simulink model "wlan_phy_rx_pmd"  (Simulink 7.8)
settings: Solver=VariableStepDiscrete  SolverMode=SingleTasking  StartTime=0.0  StopTime=rx_sim.sim_time  RelTol=1e-3
PostLoadFcn:
  | wlan_phy_rx_init
InitFcn:
  | wlan_phy_rx_init
StartFcn:
  | wlan_phy_rx_init
values from neighbouring files (symbols this model uses):
  barker_seq20 = 0.95 * circshift(barker_seq20, [0 4]) ./ max(abs(barker_seq20))    [wlan_phy_rx_init.m]
  bit_scrambler_lfsr_bytes = sum(bit_scrambler_lfsr_bytes .* repmat(2.^[0:7], 127, 1),2)    [wlan_phy_rx_init.m]
  longCorr_coef_i = [3*real(longCorr_coef)]    [wlan_phy_rx_init.m]
  longCorr_coef_q = [3*imag(longCorr_coef)]    [wlan_phy_rx_init.m]
  MAX_CP_LEN = 32    [wlan_phy_rx_init.m]
  MAX_NCBPS = NCBPS_11n_64QAM    (= 312)    [wlan_phy_rx_init.m]
  MAX_NUM_BYTES = 2^16-1    (= 65535)    [wlan_phy_rx_init.m]
  MAX_NUM_SC = 64    [wlan_phy_rx_init.m]
  MAX_NUM_SYMS = 600    [wlan_phy_rx_init.m]
  NDBPS_11a_BPSK12 = 24    [wlan_phy_rx_init.m]
  preFFT_sampBuff_numSamps = 4*MAX_NUM_SC    (= 256)    [wlan_phy_rx_init.m]
  rx_sim = struct()    [wlan_phy_rx_init.m]
  scr_ind_rev = zeros(1,128)    [wlan_phy_rx_init.m]
undefined symbols (not defined in the file or in its folder): gcbh, mcs_rom_11ag, mcs_rom_11n, wlan_rx_deinterleave_rom_gen, xlBlockLoadCallback, xlGetNormalizedPeriod, xlipgetpartsetting
libraries: xbsIndex_r4 x4943, xbsEDKLib_r4 x1
(part 3 of 28)

== system wlan_phy_rx_pmd/FCS & Pkt Buf ==
  DSSS data : Inport
  OFDM data : Inport  Port=2
  Bus Selector : BusSelector  OutputSignals=byte_valid,byte_index,byte,last_byte
  Bus Selector1 : BusSelector  OutputSignals=byte_valid,byte_index,byte,last_byte
  Bytes In : Scope  NumInputPorts=8
      settings: TimeRange=10000  YMin=0~0~0~-1~-1~-1~-1~-1  YMax=1~90~225~1~1~1~1~1  SaveName=ScopeData36
  FCS Calc : Subsystem
  Gateway Out{1..4,8..9,11..12} (8 alike) : xbsIndex_r4/Gateway Out  inherit_from_input=off  hdl_port=off  timing_constraint=None  locs_specified=off  LOCs={}  UseAsDAC=off  DACChannel='1'  block_type=gatewayout
  Pkt Buf Interface : Subsystem
  wires:
  OFDM data/1 -> Bus Selector/1, Pkt Buf Interface/2(OFDM data), FCS Calc/2(OFDM data)
  DSSS data/1 -> Bus Selector1/1, Pkt Buf Interface/1(DSSS data), FCS Calc/1(DSSS data)
  Bus Selector1/1 -> Gateway Out9/1  "<byte_valid>"
  Bus Selector1/2 -> Gateway Out8/1  "<byte_index>"
  Bus Selector1/3 -> Gateway Out11/1  "<byte>"
  Bus Selector1/4 -> Gateway Out12/1  "<last_byte>"
  Bus Selector/1 -> Gateway Out1/1  "<byte_valid>"
  Bus Selector/2 -> Gateway Out2/1  "<byte_index>"
  Bus Selector/3 -> Gateway Out3/1  "<byte>"
  Bus Selector/4 -> Gateway Out4/1  "<last_byte>"
  Gateway Out9/1 -> Bytes In/1  "DSSS Valid"
  Gateway Out8/1 -> Bytes In/2  "DSSS Byte Index"
  Gateway Out11/1 -> Bytes In/3  "DSSS Byte"
  Gateway Out12/1 -> Bytes In/4  "DSSS Last Byte"
  Gateway Out1/1 -> Bytes In/5  "OFDM Valid"
  Gateway Out2/1 -> Bytes In/6  "OFDM Byte Index"
  Gateway Out3/1 -> Bytes In/7  "OFDM Byte"
  Gateway Out4/1 -> Bytes In/8  "OFDM Last Byte"

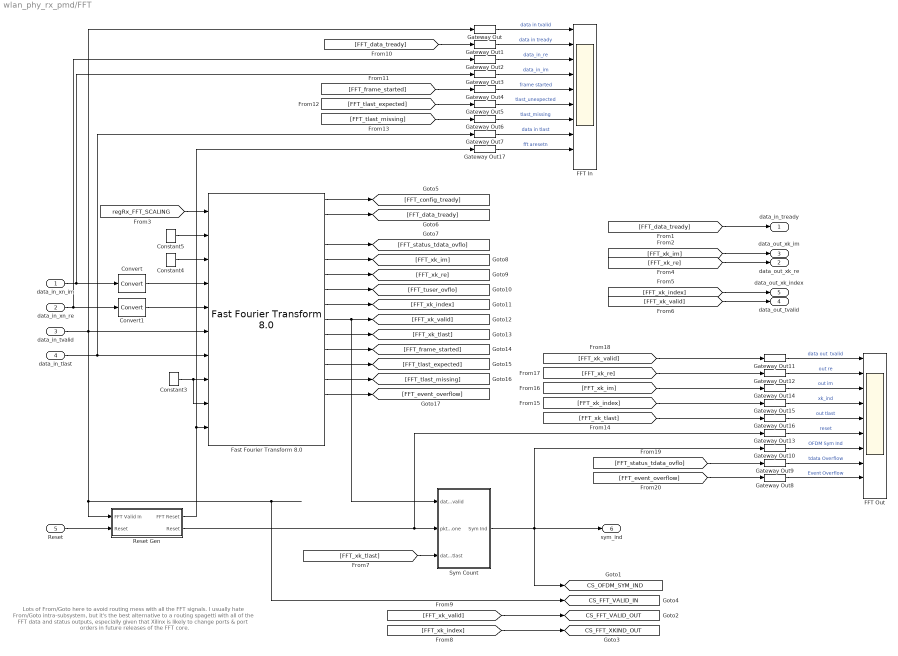
== system wlan_phy_rx_pmd/FFT ==
  note: Lots of From/Goto here to avoid routing
      | mess with all the FFT signals. I usually hate
      | From/Goto intra-subsystem, but it's the best
      | alternative to a routing spagetti with all of the
      | FFT data and status outputs, especially given
      | that Xilinx is likely to change ports & port orders
      | in future releases of the FFT core.
  data_in_xn_im : Inport
  data_in_xn_re : Inport  Port=2
  data_in_tvalid : Inport  Port=3
  data_in_tlast : Inport  Port=4
  Reset : Inport  Port=5
  Constant3 : xbsIndex_r4/Constant  const=1  gui_display_data_type=Boolean  arith_type=Boolean  n_bits=1  bin_pt=0  preci_type=Single  exp_width=8  frac_width=24  explicit_period=on  period=1  equ=P=C  opselect=C  inp2=PCIN>>17  opr=+  inp1=P  carry=CIN  dbl_ovrd=off  block_type=constant
  Constant4 : xbsIndex_r4/Constant  const=1  gui_display_data_type=Boolean  arith_type=Boolean  n_bits=1  bin_pt=0  preci_type=Single  exp_width=8  frac_width=24  explicit_period=on  period=1  equ=P=C  opselect=C  inp2=PCIN>>17  opr=+  inp1=P  carry=CIN  dbl_ovrd=off  block_type=constant
  Constant5 : xbsIndex_r4/Constant  const=1  gui_display_data_type=Fixed-point  arith_type=Unsigned  n_bits=1  bin_pt=0  preci_type=Single  exp_width=8  frac_width=24  explicit_period=on  period=1  equ=P=C  opselect=C  inp2=PCIN>>17  opr=+  inp1=P  carry=CIN  dbl_ovrd=off  block_type=constant
  Convert : xbsIndex_r4/Convert  gui_display_data_type=Fixed-point  arith_type="Signed  (2's comp)"  n_bits=24  bin_pt=23  float_type=Single  exp_bits=8  fraction_bits=24  quantization=Truncate  overflow=Wrap  en=off  latency=0  dbl_ovrd=off  pipeline=off  block_type=convert
  Convert1 : xbsIndex_r4/Convert  gui_display_data_type=Fixed-point  arith_type="Signed  (2's comp)"  n_bits=24  bin_pt=23  float_type=Single  exp_bits=8  fraction_bits=24  quantization=Truncate  overflow=Wrap  en=off  latency=0  dbl_ovrd=off  pipeline=off  block_type=convert
  FFT In : Scope  NumInputPorts=10
      settings: TimeRange=30000  YMin=-0.1~0~-0.5~-0.5~0~-1~-1~0~0~-1  YMax=1.1~1~0.5~0.5~1~1~1~1~1~1
  FFT Out : Scope  NumInputPorts=10
      settings: TimeRange=40000  YMin=0~-1~-1~0~0~0~0~0~-1~-1  YMax=1~1~1~80~1~1~40~1~1~1  SaveName=ScopeData29
  "Fast Fourier Transform 8.0 " : xbsIndex_r4/Fast Fourier Transform 8.0   transform_length=64  target_clock_frequency=160  target_data_throughput=50  implementation_options=radix_4_burst_io  run_time_configurable_transform_length=off  phase_factor_width=24  scaling_options=scaled  rounding_modes=convergent_rounding  aclken=off  aresetn=on  cyclic_prefix_insertion=off  output_ordering=natural_order  throttle_scheme=nonrealtime  xk_index=on  ovflo=on  trim_axipin_name=on  memory_options_data=block_ram  memory_options_phase_factors=block_ram  number_of_stages_using_block_ram_for_data_and_phase_factors=0  memory_options_reorder=block_ram  memory_options_hybrid=off  complex_mult_type=use_mults_performance  butterfly_type=use_luts  channels=1  input_ordering=natural_order  input_width=16  ip_name=Fast Fourier Transform  ip_version=8.0  dsptool_ready=true  ipcore_usecache=true  ipcore_useipmodelcache=true  wrapper_available=true  port_translation_map={ 'aclken' => 'en', 'aresetn' => 'rst'}  block_type=xfft_v8_0
  Gateway Out{,1..17} (18 alike) : xbsIndex_r4/Gateway Out  inherit_from_input=off  hdl_port=off  timing_constraint=None  locs_specified=off  LOCs={}  UseAsDAC=off  DACChannel='1'  block_type=gatewayout
  Reset Gen : Subsystem
  Sym Count : Subsystem
  data_in_tready : Outport
  data_out_xk_re : Outport  Port=2
  data_out_xk_im : Outport  Port=3
  data_out_tvalid : Outport  Port=4
  data_out_xk_index : Outport  Port=5
  sym_ind : Outport  Port=6
  tag scope: CS_FFT_VALID_IN=global, CS_FFT_VALID_OUT=global, CS_FFT_XKIND_OUT=global, CS_OFDM_SYM_IND=global
  wires:
  Gateway Out13/1 -> FFT Out/6  "reset"
  Gateway Out16/1 -> FFT Out/5  "out tlast"
  Gateway Out15/1 -> FFT Out/4  "xk_ind"
  Gateway Out14/1 -> FFT Out/3  "out im"
  Gateway Out12/1 -> FFT Out/2  "out re"
  Gateway Out11/1 -> FFT Out/1  "data out  tvalid"
  [FFT_xk_index] -> data_out_xk_index/1
  [FFT_xk_re] -> data_out_xk_re/1
  [FFT_xk_tlast] -> Sym Count/3(data_out_tlast)
  [FFT_xk_valid] -> data_out_tvalid/1
  [FFT_xk_im] -> data_out_xk_im/1
  [FFT_data_tready] -> data_in_tready/1
  Gateway Out7/1 -> FFT In/8  "data in tlast"
  Gateway Out6/1 -> FFT In/7  "tlast_missing"
  Gateway Out5/1 -> FFT In/6  "tlast_unexpected"
  Gateway Out4/1 -> FFT In/5  "frame started"
  Gateway Out3/1 -> FFT In/4  "data_in_im"
  Gateway Out2/1 -> FFT In/3  "data_in_re"
  Gateway Out1/1 -> FFT In/2  "data in tready"
  Gateway Out/1 -> FFT In/1  "data in tvalid"
  "Fast Fourier Transform 8.0 "/1 -> [FFT_config_tready]
  "Fast Fourier Transform 8.0 "/2 -> [FFT_data_tready]
  "Fast Fourier Transform 8.0 "/4 -> [FFT_status_tdata_ovflo]
  "Fast Fourier Transform 8.0 "/5 -> [FFT_xk_im]
  "Fast Fourier Transform 8.0 "/6 -> [FFT_xk_re]
  "Fast Fourier Transform 8.0 "/7 -> [FFT_tuser_ovflo]
  "Fast Fourier Transform 8.0 "/8 -> [FFT_xk_index]
  "Fast Fourier Transform 8.0 "/9 -> [FFT_xk_valid], Sym Count/1(data_in_tvalid)
  "Fast Fourier Transform 8.0 "/10 -> [FFT_xk_tlast]
  "Fast Fourier Transform 8.0 "/11 -> [FFT_frame_started]
  "Fast Fourier Transform 8.0 "/12 -> [FFT_tlast_expected]
  "Fast Fourier Transform 8.0 "/13 -> [FFT_tlast_missing]
  "Fast Fourier Transform 8.0 "/14 -> [FFT_event_overflow]
  Constant3/1 -> "Fast Fourier Transform 8.0 "/8, "Fast Fourier Transform 8.0 "/9
  Constant4/1 -> "Fast Fourier Transform 8.0 "/3
  Constant5/1 -> "Fast Fourier Transform 8.0 "/2
  [regRx_FFT_SCALING] -> "Fast Fourier Transform 8.0 "/1
  [FFT_xk_index] -> [CS_FFT_XKIND_OUT]
  [FFT_xk_valid] -> [CS_FFT_VALID_OUT]
  Reset Gen/2(" Reset") -> Sym Count/2(pkt_done), Gateway Out13/1
  Reset/1 -> Reset Gen/2(Reset)
  data_in_tvalid/1 -> "Fast Fourier Transform 8.0 "/6, Reset Gen/1(FFT Valid In), [CS_FFT_VALID_IN], Gateway Out/1
  Sym Count/1(Sym Ind) -> sym_ind/1, Gateway Out10/1, [CS_OFDM_SYM_IND]
  data_in_tlast/1 -> "Fast Fourier Transform 8.0 "/7, Gateway Out7/1
  data_in_xn_im/1 -> Gateway Out3/1, Convert/1
  data_in_xn_re/1 -> Gateway Out2/1, Convert1/1
  Gateway Out10/1 -> FFT Out/7  "OFDM Sym Ind"
  Gateway Out9/1 -> FFT Out/8  "tdata Overflow"
  [FFT_data_tready] -> Gateway Out1/1
  [FFT_frame_started] -> Gateway Out4/1
  [FFT_tlast_expected] -> Gateway Out5/1
  [FFT_tlast_missing] -> Gateway Out6/1
  [FFT_xk_tlast] -> Gateway Out16/1
  [FFT_xk_index] -> Gateway Out15/1
  [FFT_xk_im] -> Gateway Out14/1
  [FFT_xk_re] -> Gateway Out12/1
  [FFT_xk_valid] -> Gateway Out11/1
  [FFT_status_tdata_ovflo] -> Gateway Out9/1
  [FFT_event_overflow] -> Gateway Out8/1
  Gateway Out8/1 -> FFT Out/9  "Event Overflow"
  Reset Gen/1(FFT Reset) -> "Fast Fourier Transform 8.0 "/10, Gateway Out17/1
  Gateway Out17/1 -> FFT In/9  "fft aresetn"
  Convert/1 -> "Fast Fourier Transform 8.0 "/4
  Convert1/1 -> "Fast Fourier Transform 8.0 "/5

== system wlan_phy_rx_pmd/Inputs ==
  AGC_DONE : xbsIndex_r4/Gateway In  gui_display_data_type=Boolean  arith_type=Boolean  n_bits=5  bin_pt=0  preci_type=Single  exp_width=8  frac_width=24  quantization="Round  (unbiased: +/- Inf)"  overflow=Saturate  period=1  dbl_ovrd=off  timing_constraint=None  locs_specified=off  LOCs={}  UseAsADC=off  ADCChannel='1'  inherit_from_input=off  hdl_port=on  block_type=gatewayin
  Bus Creator{,1..3} (4 alike) : BusCreator
  Constant : Constant  Value=0
  Constant1 : Constant
  Convert2 : xbsIndex_r4/Convert  gui_display_data_type=Boolean  arith_type=Boolean  n_bits=1  bin_pt=0  float_type=Single  exp_bits=8  fraction_bits=24  quantization=Truncate  overflow=Wrap  en=off  latency=0  dbl_ovrd=off  pipeline=off  block_type=convert
  Delay3 : xbsIndex_r4/Delay  rst=off  infoeditControl=Selection of Reset will increase slice count due to use of real FFs and instead of SRLs  en=off  latency=1  dbl_ovrd=off  reg_retiming=off  block_type=delay
  Logical : xbsIndex_r4/Logical  logical_function=AND  inputs=2  en=off  latency=0  precision=Full  arith_type=Unsigned  n_bits=16  bin_pt=0  align_bp=on  dbl_ovrd=off  block_type=logical
  Pulse Generator3 : DiscretePulseGenerator  Period=160 / rx_sim.samp_rate
  RF A Inputs : Subsystem
  RF B Inputs : Subsystem
  RF C Inputs : Subsystem
  RF D Inputs : Subsystem
  RSSI Clock Gen : Subsystem
  RSSI_ADC_CLK : xbsIndex_r4/Gateway Out  inherit_from_input=off  hdl_port=on  timing_constraint=None  locs_specified=off  LOCs={}  UseAsDAC=off  DACChannel='1'  block_type=gatewayout
      settings: infoedit="Gateway out block.  Converts Xilinx fixed point inputs into ouputs of type Simulink integer, double, or fixed point.<P><P>Hardware notes:  In hardware these blocks become top level output ports or are discarded, depending on how they are configured."  block_version=8.2
  RX_IQ_SAMP_CE : xbsIndex_r4/Gateway In  gui_display_data_type=Boolean  arith_type=Boolean  n_bits=1  bin_pt=0  preci_type=Single  exp_width=8  frac_width=24  quantization="Round  (unbiased: +/- Inf)"  overflow=Saturate  period=1  dbl_ovrd=off  timing_constraint=None  locs_specified=off  LOCs={}  UseAsADC=off  ADCChannel='1'  inherit_from_input=off  hdl_port=on  block_type=gatewayin
  RX_SIGS_INVALID : xbsIndex_r4/Gateway In  gui_display_data_type=Boolean  arith_type=Boolean  n_bits=1  bin_pt=0  preci_type=Single  exp_width=8  frac_width=24  quantization="Round  (unbiased: +/- Inf)"  overflow=Saturate  period=1  dbl_ovrd=off  timing_constraint=None  locs_specified=off  LOCs={}  UseAsADC=off  ADCChannel='1'  inherit_from_input=off  hdl_port=on  block_type=gatewayin
  Register3 : xbsIndex_r4/Register  init=0  rst=off  en=off  dbl_ovrd=off  block_type=register
  Register6 : xbsIndex_r4/Register  init=0  rst=off  en=off  dbl_ovrd=off  block_type=register
  Terminator : Terminator
  negedge1 : Subsystem
  ADC I : Outport
  ADC Q : Outport  Port=2
  IQ Valid : Outport  Port=3
  RSSI : Outport  Port=4
  tag scope: AGC_DONE=global, CS_ADC_IQ_Valid=global, CS_RX_SIGS_INVALID=global
  wires:
  RSSI_ADC_CLK/1 -> Terminator/1
  RSSI Clock Gen/1(Clk) -> RSSI_ADC_CLK/1
  Constant/1 -> RX_SIGS_INVALID/1
  RX_SIGS_INVALID/1 -> Register6/1
  Logical/1 -> [CS_RX_SIGS_INVALID], RF A Inputs/1(Rst), RF B Inputs/1(Rst), RF C Inputs/1(Rst), RF D Inputs/1(Rst)
  Register6/1 -> Logical/2
  [REG_USE_RX_SIGS_INVALID_INPUT] -> Logical/1
  Bus Creator/1 -> ADC I/1
  Bus Creator1/1 -> ADC Q/1
  Bus Creator2/1 -> RSSI/1
  [RFA_ADC_I] -> Bus Creator/1  "RFA_I"
  [RFB_ADC_I] -> Bus Creator/2  "RFB_I"
  [RFA_ADC_Q] -> Bus Creator1/1  "RFA_Q"
  [RFB_ADC_Q] -> Bus Creator1/2  "RFB_Q"
  [RFA_RSSI] -> Bus Creator2/1  "RFA_RSSI"
  [RFB_RSSI] -> Bus Creator2/2  "RFB_RSSI"
  AGC_DONE/1 -> Delay3/1
  Delay3/1 -> [AGC_DONE]
  Bus Creator3/1 -> IQ Valid/1
  Convert2/1 -> [CS_ADC_IQ_Valid], RF A Inputs/2(IQ Valid), Bus Creator3/1, Bus Creator3/2, Bus Creator3/3, Bus Creator3/4, RF B Inputs/2(IQ Valid), RF C Inputs/2(IQ Valid), RF D Inputs/2(IQ Valid)
  negedge1/1(Q) -> Convert2/1
  Register3/1 -> negedge1/1(D)
  RX_IQ_SAMP_CE/1 -> Register3/1
  Pulse Generator3/1 -> RX_IQ_SAMP_CE/1
  RF A Inputs/1(ADC I) -> [RFA_ADC_I]
  RF A Inputs/2(ADC Q) -> [RFA_ADC_Q]
  RF A Inputs/3(Avg RSSI) -> [RFA_RSSI]
  RF B Inputs/1(ADC I) -> [RFB_ADC_I]
  RF B Inputs/2(ADC Q) -> [RFB_ADC_Q]
  RF B Inputs/3(Avg RSSI) -> [RFB_RSSI]
  [RFD_ADC_I] -> Bus Creator/4  "RFD_I"
  [RFC_ADC_I] -> Bus Creator/3  "RFC_I"
  [RFC_ADC_Q] -> Bus Creator1/3  "RFC_Q"
  [RFD_ADC_Q] -> Bus Creator1/4  "RFD_Q"
  [RFC_RSSI] -> Bus Creator2/3  "RFC_RSSI"
  [RFD_RSSI] -> Bus Creator2/4  "RFD_RSSI"
  RF C Inputs/1(ADC I) -> [RFC_ADC_I]
  RF C Inputs/2(ADC Q) -> [RFC_ADC_Q]
  RF C Inputs/3(Avg RSSI) -> [RFC_RSSI]
  RF D Inputs/1(ADC I) -> [RFD_ADC_I]
  RF D Inputs/2(ADC Q) -> [RFD_ADC_Q]
  RF D Inputs/3(Avg RSSI) -> [RFD_RSSI]
  Constant1/1 -> AGC_DONE/1

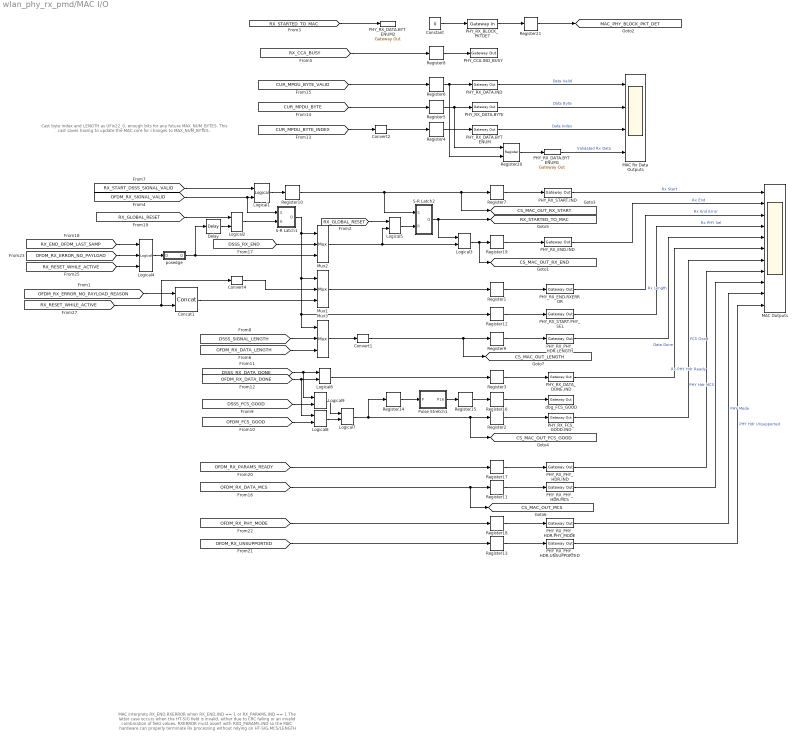
== system wlan_phy_rx_pmd/MAC I/O ==
  note: Cast byte index and LENGTH as UFix22_0, enough bits
      | for any future MAX_NUM_BYTES. This cast saves having to
      | update the MAC core for changes to MAX_NUM_BYTES.
  note: MAC interprets RX_END.RXERROR when
      | RX_END.IND == 1 or RX_PARAMS.IND == 1
      | The latter case occurs when the HT-SIG field is
      | invalid, either due to CRC failing or an invalid
      | combination of field values. RXERROR must assert
      | with RXD_PARAMS.IND so the MAC hardware can
      | properly terminate Rx processing without relying on
      | HT-SIG.MCS/LENGTH
  Concat1 : xbsIndex_r4/Concat  num_inputs=2  dbl_ovrd=off  block_type=concat
  Constant : Constant  Value=0
  Convert1 : xbsIndex_r4/Convert  gui_display_data_type=Fixed-point  arith_type=Unsigned  n_bits=ceil(log2(MAX_NUM_BYTES))  bin_pt=0  float_type=Single  exp_bits=8  fraction_bits=24  quantization=Truncate  overflow=Wrap  en=off  latency=0  dbl_ovrd=off  pipeline=off  block_type=convert
  Convert2 : xbsIndex_r4/Convert  gui_display_data_type=Fixed-point  arith_type=Unsigned  n_bits=ceil(log2(MAX_NUM_BYTES))  bin_pt=0  float_type=Single  exp_bits=8  fraction_bits=24  quantization=Truncate  overflow=Wrap  en=off  latency=0  dbl_ovrd=off  pipeline=off  block_type=convert
  Convert4 : xbsIndex_r4/Convert  gui_display_data_type=Fixed-point  arith_type=Unsigned  n_bits=1  bin_pt=0  float_type=Single  exp_bits=8  fraction_bits=24  quantization=Truncate  overflow=Wrap  en=off  latency=0  dbl_ovrd=off  pipeline=off  block_type=convert
  Delay : xbsIndex_r4/Delay  rst=off  infoeditControl=Selection of Reset will increase slice count due to use of real FFs and instead of SRLs  en=off  latency=8  dbl_ovrd=off  reg_retiming=off  block_type=delay
  Logical{1..2,5..7} (5 alike) : xbsIndex_r4/Logical  logical_function=OR  inputs=2  en=off  latency=0  precision=Full  arith_type=Unsigned  n_bits=16  bin_pt=0  align_bp=on  dbl_ovrd=off  block_type=logical
  Logical3 : xbsIndex_r4/Logical  logical_function=AND  inputs=2  en=off  latency=0  precision=Full  arith_type=Unsigned  n_bits=16  bin_pt=0  align_bp=on  dbl_ovrd=off  block_type=logical
  Logical4 : xbsIndex_r4/Logical  logical_function=OR  inputs=3  en=off  latency=0  precision=Full  arith_type=Unsigned  n_bits=16  bin_pt=0  align_bp=on  dbl_ovrd=off  block_type=logical
  Logical8 : xbsIndex_r4/Logical  logical_function=AND  inputs=2  en=off  latency=0  precision=Full  arith_type=Unsigned  n_bits=16  bin_pt=0  align_bp=on  dbl_ovrd=off  block_type=logical
  Logical9 : xbsIndex_r4/Logical  logical_function=AND  inputs=2  en=off  latency=0  precision=Full  arith_type=Unsigned  n_bits=16  bin_pt=0  align_bp=on  dbl_ovrd=off  block_type=logical
  MAC Outputs : Scope  NumInputPorts=11
      settings: TimeRange=21436  YMin=-1~-1~-1~-1~133~-1~-1~-1~-1~1~-1  YMax=1~1~1~1~133~1~1~1~1~1~1  SaveName=ScopeData37
  MAC Rx Data Outputs : Scope  NumInputPorts=4
      settings: TimeRange=32908  YMin=0~0~0~0  YMax=1~275~1100~275  SaveName=ScopeData32
  Mux1 : xbsIndex_r4/Mux  inputs=2  en=off  latency=0  precision=Full  arith_type=Unsigned  n_bits=16  bin_pt=14  quantization=Truncate  overflow=Wrap  dbl_ovrd=off  block_type=mux
  Mux2 : xbsIndex_r4/Mux  inputs=2  en=off  latency=0  precision=Full  arith_type=Unsigned  n_bits=16  bin_pt=14  quantization=Truncate  overflow=Wrap  dbl_ovrd=off  block_type=mux
  Mux3 : xbsIndex_r4/Mux  inputs=2  en=off  latency=0  precision=Full  arith_type=Unsigned  n_bits=16  bin_pt=14  quantization=Truncate  overflow=Wrap  dbl_ovrd=off  block_type=mux
  PHY_CCA.IND_BUSY : xbsIndex_r4/Gateway Out  inherit_from_input=off  hdl_port=on  timing_constraint=None  locs_specified=off  LOCs={}  UseAsDAC=off  DACChannel='1'  block_type=gatewayout
  PHY_RX_BLOCK_PKTDET : xbsIndex_r4/Gateway In  gui_display_data_type=Boolean  arith_type=Boolean  n_bits=16  bin_pt=14  preci_type=Single  exp_width=8  frac_width=24  quantization="Round  (unbiased: +/- Inf)"  overflow=Saturate  period=1  dbl_ovrd=off  timing_constraint=None  locs_specified=off  LOCs={}  UseAsADC=off  ADCChannel='1'  inherit_from_input=off  hdl_port=on  block_type=gatewayin
  PHY_RX_DATA.BYTE : xbsIndex_r4/Gateway Out  inherit_from_input=off  hdl_port=on  timing_constraint=None  locs_specified=off  LOCs={}  UseAsDAC=off  DACChannel='1'  block_type=gatewayout
  PHY_RX_DATA.BYTENUM : xbsIndex_r4/Gateway Out  inherit_from_input=off  hdl_port=on  timing_constraint=None  locs_specified=off  LOCs={}  UseAsDAC=off  DACChannel='1'  block_type=gatewayout
  PHY_RX_DATA.BYTENUM1 : xbsIndex_r4/Gateway Out  inherit_from_input=off  hdl_port=off  timing_constraint=None  locs_specified=off  LOCs={}  UseAsDAC=off  DACChannel='1'  block_type=gatewayout
  PHY_RX_DATA.BYTENUM2 : xbsIndex_r4/Gateway Out  inherit_from_input=off  hdl_port=off  timing_constraint=None  locs_specified=off  LOCs={}  UseAsDAC=off  DACChannel='1'  block_type=gatewayout
  PHY_RX_DATA.IND : xbsIndex_r4/Gateway Out  inherit_from_input=off  hdl_port=on  timing_constraint=None  locs_specified=off  LOCs={}  UseAsDAC=off  DACChannel='1'  block_type=gatewayout
  PHY_RX_DATA_DONE.IND : xbsIndex_r4/Gateway Out  inherit_from_input=off  hdl_port=on  timing_constraint=None  locs_specified=off  LOCs={}  UseAsDAC=off  DACChannel='1'  block_type=gatewayout
  PHY_RX_END.IND : xbsIndex_r4/Gateway Out  inherit_from_input=off  hdl_port=on  timing_constraint=None  locs_specified=off  LOCs={}  UseAsDAC=off  DACChannel='1'  block_type=gatewayout
  PHY_RX_END.RXERROR : xbsIndex_r4/Gateway Out  inherit_from_input=off  hdl_port=on  timing_constraint=None  locs_specified=off  LOCs={}  UseAsDAC=off  DACChannel='1'  block_type=gatewayout
  PHY_RX_FCS_GOOD.IND : xbsIndex_r4/Gateway Out  inherit_from_input=off  hdl_port=on  timing_constraint=None  locs_specified=off  LOCs={}  UseAsDAC=off  DACChannel='1'  block_type=gatewayout
  PHY_RX_PHY_HDR.IND : xbsIndex_r4/Gateway Out  inherit_from_input=off  hdl_port=on  timing_constraint=None  locs_specified=off  LOCs={}  UseAsDAC=off  DACChannel='1'  block_type=gatewayout
  PHY_RX_PHY_HDR.LENGTH : xbsIndex_r4/Gateway Out  inherit_from_input=off  hdl_port=on  timing_constraint=None  locs_specified=off  LOCs={}  UseAsDAC=off  DACChannel='1'  block_type=gatewayout
  PHY_RX_PHY_HDR.MCS : xbsIndex_r4/Gateway Out  inherit_from_input=off  hdl_port=on  timing_constraint=None  locs_specified=off  LOCs={}  UseAsDAC=off  DACChannel='1'  block_type=gatewayout
  PHY_RX_PHY_HDR.PHY_MODE : xbsIndex_r4/Gateway Out  inherit_from_input=off  hdl_port=on  timing_constraint=None  locs_specified=off  LOCs={}  UseAsDAC=off  DACChannel='1'  block_type=gatewayout
  PHY_RX_PHY_HDR.UNSUPPORTED : xbsIndex_r4/Gateway Out  inherit_from_input=off  hdl_port=on  timing_constraint=None  locs_specified=off  LOCs={}  UseAsDAC=off  DACChannel='1'  block_type=gatewayout
  PHY_RX_START.IND : xbsIndex_r4/Gateway Out  inherit_from_input=off  hdl_port=on  timing_constraint=None  locs_specified=off  LOCs={}  UseAsDAC=off  DACChannel='1'  block_type=gatewayout
  PHY_RX_START.PHY_SEL : xbsIndex_r4/Gateway Out  inherit_from_input=off  hdl_port=on  timing_constraint=None  locs_specified=off  LOCs={}  UseAsDAC=off  DACChannel='1'  block_type=gatewayout
  Pulse Stretch1 : Subsystem
  Register{1..19,21} (20 alike) : xbsIndex_r4/Register  init=0  rst=off  en=off  dbl_ovrd=off  block_type=register
  Register20 : xbsIndex_r4/Register  init=0  rst=off  en=on  dbl_ovrd=off  block_type=register
  S-R Latch1 : Subsystem
  S-R Latch2 : Subsystem
  dbg_FCS_GOOD : xbsIndex_r4/Gateway Out  inherit_from_input=off  hdl_port=on  timing_constraint=None  locs_specified=off  LOCs={}  UseAsDAC=off  DACChannel='1'  block_type=gatewayout
  posedge : Subsystem
  tag scope: CS_MAC_OUT_FCS_GOOD=global, CS_MAC_OUT_LENGTH=global, CS_MAC_OUT_MCS=global, CS_MAC_OUT_RX_END=global, CS_MAC_OUT_RX_START=global, MAC_PHY_BLOCK_PKT_DET=global, RX_STARTED_TO_MAC=global
  wires:
  Register1/1 -> PHY_RX_END.RXERROR/1
  Register2/1 -> PHY_RX_FCS_GOOD.IND/1
  Register3/1 -> PHY_RX_DATA_DONE.IND/1
  [CUR_MPDU_BYTE] -> Register5/1
  [CUR_MPDU_BYTE_VALID] -> Register6/1
  [CUR_MPDU_BYTE_INDEX] -> Convert2/1
  Register4/1 -> PHY_RX_DATA.BYTENUM/1
  Register5/1 -> PHY_RX_DATA.BYTE/1, Register20/1
  Register6/1 -> PHY_RX_DATA.IND/1, Register20/2
  [RX_CCA_BUSY] -> Register8/1
  Register7/1 -> PHY_RX_START.IND/1
  Register8/1 -> PHY_CCA.IND_BUSY/1
  Register9/1 -> PHY_RX_PHY_HDR.LENGTH/1
  [RX_START_DSSS_SIGNAL_VALID] -> Logical1/1
  [OFDM_RX_SIGNAL_VALID] -> Logical1/2, S-R Latch1/1(S)
  Logical1/1 -> Register10/1
  [OFDM_RX_DATA_LENGTH] -> Mux3/3
  [DSSS_SIGNAL_LENGTH] -> Mux3/2
  Mux3/1 -> Convert1/1
  Mux1/1 -> Register1/1
  [DSSS_RX_END] -> Mux2/2
  Logical7/1 -> Register14/1, Register2/1, [CS_MAC_OUT_FCS_GOOD]
  Register10/1 -> S-R Latch2/1(S), Register7/1, [CS_MAC_OUT_RX_START]
  Register14/1 -> Pulse Stretch1/1(P)
  Register16/1 -> dbg_FCS_GOOD/1
  S-R Latch2/1(Q) -> [RX_STARTED_TO_MAC], Logical3/1
  Convert2/1 -> Register4/1
  Register12/1 -> PHY_RX_START.PHY_SEL/1
  Convert1/1 -> Register9/1, [CS_MAC_OUT_LENGTH]
  PHY_RX_DATA.IND/1 -> MAC Rx Data Outputs/1  "Data Valid"
  PHY_RX_DATA.BYTE/1 -> MAC Rx Data Outputs/2  "Data Byte"
  PHY_RX_DATA.BYTENUM/1 -> MAC Rx Data Outputs/3  "Data Index"
  Register20/1 -> PHY_RX_DATA.BYTENUM1/1
  PHY_RX_DATA.BYTENUM1/1 -> MAC Rx Data Outputs/4  "Validated Rx Data"
  S-R Latch1/1(Q) -> Mux2/1, Register12/1, Mux3/1, Mux1/1
  Logical5/1 -> S-R Latch2/2(R)
  Logical2/1 -> S-R Latch1/2(R)
  [RX_GLOBAL_RESET] -> Logical2/1
  Mux2/1 -> Logical5/2, Logical3/2
  Register19/1 -> PHY_RX_END.IND/1
  [RX_GLOBAL_RESET] -> Logical5/1
  Logical3/1 -> Register19/1, [CS_MAC_OUT_RX_END]
  Register15/1 -> Register16/1
  Pulse Stretch1/1(P16) -> Register15/1
  Register11/1 -> PHY_RX_PHY_HDR.MCS/1
  [OFDM_RX_DATA_MCS] -> Register11/1, [CS_MAC_OUT_MCS]
  Register13/1 -> PHY_RX_PHY_HDR.UNSUPPORTED/1
  [OFDM_RX_UNSUPPORTED] -> Register13/1
  [OFDM_RX_PARAMS_READY] -> Register17/1
  Register17/1 -> PHY_RX_PHY_HDR.IND/1
  Register18/1 -> PHY_RX_PHY_HDR.PHY_MODE/1
  [OFDM_RX_PHY_MODE] -> Register18/1
  [RX_END_OFDM_LAST_SAMP] -> Logical4/1
  [OFDM_RX_ERROR_NO_PAYLOAD] -> Logical4/2
  [RX_RESET_WHILE_ACTIVE] -> Logical4/3
  Logical4/1 -> posedge/1(D)
  [OFDM_RX_ERROR_NO_PAYLOAD_REASON] -> Concat1/1
  [RX_RESET_WHILE_ACTIVE] -> Concat1/2, Convert4/1
  Concat1/1 -> Mux1/3
  Delay/1 -> Logical2/2
  Convert4/1 -> Mux1/2
  PHY_RX_START.IND/1 -> MAC Outputs/1  "Rx Start"
  PHY_RX_END.IND/1 -> MAC Outputs/2  "Rx End"
  PHY_RX_END.RXERROR/1 -> MAC Outputs/3  "Rx End Error"
  PHY_RX_START.PHY_SEL/1 -> MAC Outputs/4  "Rx PHY Sel"
  PHY_RX_PHY_HDR.LENGTH/1 -> MAC Outputs/5  "Rx Length"
  PHY_RX_DATA_DONE.IND/1 -> MAC Outputs/6  "Data Done"
  PHY_RX_FCS_GOOD.IND/1 -> MAC Outputs/7  "FCS Good"
  PHY_RX_PHY_HDR.IND/1 -> MAC Outputs/8  "Rx PHY Hdr Ready"
  PHY_RX_PHY_HDR.MCS/1 -> MAC Outputs/9  "PHY Hdr MCS"
  PHY_RX_PHY_HDR.PHY_MODE/1 -> MAC Outputs/10  "PHY Mode"
  PHY_RX_PHY_HDR.UNSUPPORTED/1 -> MAC Outputs/11  "PHY Hdr Unsupported"
  Constant/1 -> PHY_RX_BLOCK_PKTDET/1
  PHY_RX_BLOCK_PKTDET/1 -> Register21/1
  Register21/1 -> [MAC_PHY_BLOCK_PKT_DET]
  [RX_STARTED_TO_MAC] -> PHY_RX_DATA.BYTENUM2/1
  posedge/1(Q) -> Mux2/3, Delay/1
  [OFDM_RX_DATA_DONE] -> Logical6/2, Logical8/1
  [DSSS_RX_DATA_DONE] -> Logical6/1, Logical9/1
  Logical8/1 -> Logical7/2
  [OFDM_FCS_GOOD] -> Logical8/2
  Logical9/1 -> Logical7/1
  [DSSS_FCS_GOOD] -> Logical9/2
  Logical6/1 -> Register3/1
